simulink library "Garbage"  (R2023a)
settings: Solver=ode45  StartTime=0.0  StopTime=10.0  RelTol=1e-3  EnableMultiTasking=on
undefined symbols (not defined in the file or in its folder): c, ending_column_index, ending_row_index, framebuffer_16x1, framebuffer_2x2, framebuffer_4x4, framebuffer_8x8, HEIGHT, hot, POS_X, POS_Y, starting_column_index, starting_row_index, WIDTH
libraries: Memory_Blocks_Lib x459, simulink x235, simulink_extras x132, dspmtrx3 x116, Bit_Multiplexers_Lib x67, Bit_Demultiplexers_Lib x49, Byte_Multiplexers_Lib x40, Miscellaneous x38, Computer_Library x17, Decoders_Lib x14, commutil2 x12, Arithmetic_Operations_Lib x8
(part 24 of 26)

== system Garbage/Text_Display_1x16/Character_Table/Characters(ARROW_DOWN..RECTANGLE) ==
  Address_3_In(1b) : Inport
  Address_2_In(1b) : Inport  Port=2
  Address_1_In(1b) : Inport  Port=3
  Address_0_In(1b) : Inport  Port=4
  Byte_Multiplexer_16_to_1(Byte_0) : Byte_Multiplexers_Lib/Byte_Multiplexers_Lib/Byte_Multiplexer_16_to_1
  Byte_Multiplexer_16_to_1(Byte_1) : Byte_Multiplexers_Lib/Byte_Multiplexers_Lib/Byte_Multiplexer_16_to_1
  Byte_Multiplexer_16_to_1(Byte_2) : Byte_Multiplexers_Lib/Byte_Multiplexers_Lib/Byte_Multiplexer_16_to_1
  Byte_Multiplexer_16_to_1(Byte_3) : Byte_Multiplexers_Lib/Byte_Multiplexers_Lib/Byte_Multiplexer_16_to_1
  Byte_Multiplexer_16_to_1(Byte_4) : Byte_Multiplexers_Lib/Byte_Multiplexers_Lib/Byte_Multiplexer_16_to_1
  Byte_Multiplexer_16_to_1(Byte_5) : Byte_Multiplexers_Lib/Byte_Multiplexers_Lib/Byte_Multiplexer_16_to_1
  Byte_Multiplexer_16_to_1(Byte_6) : Byte_Multiplexers_Lib/Byte_Multiplexers_Lib/Byte_Multiplexer_16_to_1
  Byte_Multiplexer_16_to_1(Byte_7) : Byte_Multiplexers_Lib/Byte_Multiplexers_Lib/Byte_Multiplexer_16_to_1
  Char_ARROW_DOWN : Subsystem
  Char_ARROW_LEFT : Subsystem
  Char_ARROW_RIGHT : Subsystem
  Char_COMMA : Subsystem
  Char_CURSOR : Subsystem
  Char_DOT : Subsystem
  Char_EXCLAMATION_MARK : Subsystem
  Char_LEFT_PARENTHESES : Subsystem
  Char_PERCENT : Subsystem
  Char_QUESTION_MARK : Subsystem
  Char_QUOTE : Subsystem
  Char_RECTANGLE : Subsystem
  Char_RIGHT_PARENTHESES : Subsystem
  Char_SHARP : Subsystem
  Char_SPACE : Subsystem
  Char_UNDERSCORE : Subsystem
  Row_7(1B) : Outport
  Row_6(1B) : Outport  Port=2
  Row_5(1B) : Outport  Port=3
  Row_4(1B) : Outport  Port=4
  Row_3(1B) : Outport  Port=5
  Row_2(1B) : Outport  Port=6
  Row_1(1B) : Outport  Port=7
  Row_0(1B) : Outport  Port=8
  wires:
  Address_3_In(1b)/1 -> Byte_Multiplexer_16_to_1(Byte_0)/1, Byte_Multiplexer_16_to_1(Byte_1)/1, Byte_Multiplexer_16_to_1(Byte_2)/1, Byte_Multiplexer_16_to_1(Byte_3)/1, Byte_Multiplexer_16_to_1(Byte_4)/1, Byte_Multiplexer_16_to_1(Byte_5)/1, Byte_Multiplexer_16_to_1(Byte_6)/1, Byte_Multiplexer_16_to_1(Byte_7)/1
  Address_2_In(1b)/1 -> Byte_Multiplexer_16_to_1(Byte_0)/2, Byte_Multiplexer_16_to_1(Byte_1)/2, Byte_Multiplexer_16_to_1(Byte_2)/2, Byte_Multiplexer_16_to_1(Byte_3)/2, Byte_Multiplexer_16_to_1(Byte_4)/2, Byte_Multiplexer_16_to_1(Byte_5)/2, Byte_Multiplexer_16_to_1(Byte_6)/2, Byte_Multiplexer_16_to_1(Byte_7)/2
  Address_1_In(1b)/1 -> Byte_Multiplexer_16_to_1(Byte_0)/3, Byte_Multiplexer_16_to_1(Byte_1)/3, Byte_Multiplexer_16_to_1(Byte_2)/3, Byte_Multiplexer_16_to_1(Byte_3)/3, Byte_Multiplexer_16_to_1(Byte_4)/3, Byte_Multiplexer_16_to_1(Byte_5)/3, Byte_Multiplexer_16_to_1(Byte_6)/3, Byte_Multiplexer_16_to_1(Byte_7)/3
  Address_0_In(1b)/1 -> Byte_Multiplexer_16_to_1(Byte_0)/4, Byte_Multiplexer_16_to_1(Byte_1)/4, Byte_Multiplexer_16_to_1(Byte_2)/4, Byte_Multiplexer_16_to_1(Byte_3)/4, Byte_Multiplexer_16_to_1(Byte_4)/4, Byte_Multiplexer_16_to_1(Byte_5)/4, Byte_Multiplexer_16_to_1(Byte_6)/4, Byte_Multiplexer_16_to_1(Byte_7)/4
  Byte_Multiplexer_16_to_1(Byte_0)/1 -> Row_0(1B)/1
  Byte_Multiplexer_16_to_1(Byte_1)/1 -> Row_1(1B)/1
  Byte_Multiplexer_16_to_1(Byte_2)/1 -> Row_2(1B)/1
  Byte_Multiplexer_16_to_1(Byte_3)/1 -> Row_3(1B)/1
  Byte_Multiplexer_16_to_1(Byte_4)/1 -> Row_4(1B)/1
  Byte_Multiplexer_16_to_1(Byte_5)/1 -> Row_5(1B)/1
  Byte_Multiplexer_16_to_1(Byte_6)/1 -> Row_6(1B)/1
  Byte_Multiplexer_16_to_1(Byte_7)/1 -> Row_7(1B)/1
  Char_RECTANGLE/8(Row_0(1B)) -> Byte_Multiplexer_16_to_1(Byte_0)/5
  Char_CURSOR/8(Row_0(1B)) -> Byte_Multiplexer_16_to_1(Byte_0)/6
  Char_SPACE/8(Row_0(1B)) -> Byte_Multiplexer_16_to_1(Byte_0)/7
  Char_RIGHT_PARENTHESES/8(Row_0(1B)) -> Byte_Multiplexer_16_to_1(Byte_0)/8
  Char_LEFT_PARENTHESES/8(Row_0(1B)) -> Byte_Multiplexer_16_to_1(Byte_0)/9
  Char_PERCENT/8(Row_0(1B)) -> Byte_Multiplexer_16_to_1(Byte_0)/10
  Char_SHARP/8(Row_0(1B)) -> Byte_Multiplexer_16_to_1(Byte_0)/11
  Char_QUOTE/8(Row_0(1B)) -> Byte_Multiplexer_16_to_1(Byte_0)/12
  Char_EXCLAMATION_MARK/8(Row_0(1B)) -> Byte_Multiplexer_16_to_1(Byte_0)/13
  Char_QUESTION_MARK/8(Row_0(1B)) -> Byte_Multiplexer_16_to_1(Byte_0)/14
  Char_COMMA/8(Row_0(1B)) -> Byte_Multiplexer_16_to_1(Byte_0)/15
  Char_DOT/8(Row_0(1B)) -> Byte_Multiplexer_16_to_1(Byte_0)/16
  Char_UNDERSCORE/8(Row_0(1B)) -> Byte_Multiplexer_16_to_1(Byte_0)/17
  Char_ARROW_RIGHT/8(Row_0(1B)) -> Byte_Multiplexer_16_to_1(Byte_0)/18
  Char_ARROW_LEFT/8(Row_0(1B)) -> Byte_Multiplexer_16_to_1(Byte_0)/19
  Char_ARROW_DOWN/8(Row_0(1B)) -> Byte_Multiplexer_16_to_1(Byte_0)/20
  Char_RECTANGLE/7(Row_1(1B)) -> Byte_Multiplexer_16_to_1(Byte_1)/5
  Char_CURSOR/7(Row_1(1B)) -> Byte_Multiplexer_16_to_1(Byte_1)/6
  Char_SPACE/7(Row_1(1B)) -> Byte_Multiplexer_16_to_1(Byte_1)/7
  Char_LEFT_PARENTHESES/7(Row_1(1B)) -> Byte_Multiplexer_16_to_1(Byte_1)/9
  Char_PERCENT/7(Row_1(1B)) -> Byte_Multiplexer_16_to_1(Byte_1)/10
  Char_SHARP/7(Row_1(1B)) -> Byte_Multiplexer_16_to_1(Byte_1)/11
  Char_QUOTE/7(Row_1(1B)) -> Byte_Multiplexer_16_to_1(Byte_1)/12
  Char_EXCLAMATION_MARK/7(Row_1(1B)) -> Byte_Multiplexer_16_to_1(Byte_1)/13
  Char_QUESTION_MARK/7(Row_1(1B)) -> Byte_Multiplexer_16_to_1(Byte_1)/14
  Char_COMMA/7(Row_1(1B)) -> Byte_Multiplexer_16_to_1(Byte_1)/15
  Char_DOT/7(Row_1(1B)) -> Byte_Multiplexer_16_to_1(Byte_1)/16
  Char_UNDERSCORE/7(Row_1(1B)) -> Byte_Multiplexer_16_to_1(Byte_1)/17
  Char_ARROW_RIGHT/7(Row_1(1B)) -> Byte_Multiplexer_16_to_1(Byte_1)/18
  Char_ARROW_LEFT/7(Row_1(1B)) -> Byte_Multiplexer_16_to_1(Byte_1)/19
  Char_ARROW_DOWN/7(Row_1(1B)) -> Byte_Multiplexer_16_to_1(Byte_1)/20
  Char_RECTANGLE/6(Row_2(1B)) -> Byte_Multiplexer_16_to_1(Byte_2)/5
  Char_CURSOR/6(Row_2(1B)) -> Byte_Multiplexer_16_to_1(Byte_2)/6
  Char_SPACE/6(Row_2(1B)) -> Byte_Multiplexer_16_to_1(Byte_2)/7
  Char_RIGHT_PARENTHESES/6(Row_2(1B)) -> Byte_Multiplexer_16_to_1(Byte_2)/8
  Char_RIGHT_PARENTHESES/7(Row_1(1B)) -> Byte_Multiplexer_16_to_1(Byte_1)/8
  Char_LEFT_PARENTHESES/6(Row_2(1B)) -> Byte_Multiplexer_16_to_1(Byte_2)/9
  Char_PERCENT/6(Row_2(1B)) -> Byte_Multiplexer_16_to_1(Byte_2)/10
  Char_SHARP/6(Row_2(1B)) -> Byte_Multiplexer_16_to_1(Byte_2)/11
  Char_QUOTE/6(Row_2(1B)) -> Byte_Multiplexer_16_to_1(Byte_2)/12
  Char_EXCLAMATION_MARK/6(Row_2(1B)) -> Byte_Multiplexer_16_to_1(Byte_2)/13
  Char_QUESTION_MARK/6(Row_2(1B)) -> Byte_Multiplexer_16_to_1(Byte_2)/14
  Char_COMMA/6(Row_2(1B)) -> Byte_Multiplexer_16_to_1(Byte_2)/15
  Char_DOT/6(Row_2(1B)) -> Byte_Multiplexer_16_to_1(Byte_2)/16
  Char_UNDERSCORE/6(Row_2(1B)) -> Byte_Multiplexer_16_to_1(Byte_2)/17
  Char_ARROW_RIGHT/6(Row_2(1B)) -> Byte_Multiplexer_16_to_1(Byte_2)/18
  Char_ARROW_LEFT/6(Row_2(1B)) -> Byte_Multiplexer_16_to_1(Byte_2)/19
  Char_ARROW_DOWN/6(Row_2(1B)) -> Byte_Multiplexer_16_to_1(Byte_2)/20
  Char_RECTANGLE/5(Row_3(1B)) -> Byte_Multiplexer_16_to_1(Byte_3)/5
  Char_CURSOR/5(Row_3(1B)) -> Byte_Multiplexer_16_to_1(Byte_3)/6
  Char_SPACE/5(Row_3(1B)) -> Byte_Multiplexer_16_to_1(Byte_3)/7
  Char_RIGHT_PARENTHESES/5(Row_3(1B)) -> Byte_Multiplexer_16_to_1(Byte_3)/8
  Char_LEFT_PARENTHESES/5(Row_3(1B)) -> Byte_Multiplexer_16_to_1(Byte_3)/9
  Char_PERCENT/5(Row_3(1B)) -> Byte_Multiplexer_16_to_1(Byte_3)/10
  Char_SHARP/5(Row_3(1B)) -> Byte_Multiplexer_16_to_1(Byte_3)/11
  Char_QUOTE/5(Row_3(1B)) -> Byte_Multiplexer_16_to_1(Byte_3)/12
  Char_QUESTION_MARK/5(Row_3(1B)) -> Byte_Multiplexer_16_to_1(Byte_3)/14
  Char_EXCLAMATION_MARK/5(Row_3(1B)) -> Byte_Multiplexer_16_to_1(Byte_3)/13
  Char_COMMA/5(Row_3(1B)) -> Byte_Multiplexer_16_to_1(Byte_3)/15
  Char_DOT/5(Row_3(1B)) -> Byte_Multiplexer_16_to_1(Byte_3)/16
  Char_UNDERSCORE/5(Row_3(1B)) -> Byte_Multiplexer_16_to_1(Byte_3)/17
  Char_ARROW_RIGHT/5(Row_3(1B)) -> Byte_Multiplexer_16_to_1(Byte_3)/18
  Char_ARROW_LEFT/5(Row_3(1B)) -> Byte_Multiplexer_16_to_1(Byte_3)/19
  Char_ARROW_DOWN/5(Row_3(1B)) -> Byte_Multiplexer_16_to_1(Byte_3)/20
  Char_RECTANGLE/4(Row_4(1B)) -> Byte_Multiplexer_16_to_1(Byte_4)/5
  Char_CURSOR/4(Row_4(1B)) -> Byte_Multiplexer_16_to_1(Byte_4)/6
  Char_SPACE/4(Row_4(1B)) -> Byte_Multiplexer_16_to_1(Byte_4)/7
  Char_RIGHT_PARENTHESES/4(Row_4(1B)) -> Byte_Multiplexer_16_to_1(Byte_4)/8
  Char_LEFT_PARENTHESES/4(Row_4(1B)) -> Byte_Multiplexer_16_to_1(Byte_4)/9
  Char_PERCENT/4(Row_4(1B)) -> Byte_Multiplexer_16_to_1(Byte_4)/10
  Char_SHARP/4(Row_4(1B)) -> Byte_Multiplexer_16_to_1(Byte_4)/11
  Char_QUOTE/4(Row_4(1B)) -> Byte_Multiplexer_16_to_1(Byte_4)/12
  Char_EXCLAMATION_MARK/4(Row_4(1B)) -> Byte_Multiplexer_16_to_1(Byte_4)/13
  Char_QUESTION_MARK/4(Row_4(1B)) -> Byte_Multiplexer_16_to_1(Byte_4)/14
  Char_COMMA/4(Row_4(1B)) -> Byte_Multiplexer_16_to_1(Byte_4)/15
  Char_DOT/4(Row_4(1B)) -> Byte_Multiplexer_16_to_1(Byte_4)/16
  Char_UNDERSCORE/4(Row_4(1B)) -> Byte_Multiplexer_16_to_1(Byte_4)/17
  Char_ARROW_RIGHT/4(Row_4(1B)) -> Byte_Multiplexer_16_to_1(Byte_4)/18
  Char_ARROW_LEFT/4(Row_4(1B)) -> Byte_Multiplexer_16_to_1(Byte_4)/19
  Char_ARROW_DOWN/4(Row_4(1B)) -> Byte_Multiplexer_16_to_1(Byte_4)/20
  Char_RECTANGLE/3(Row_5(1B)) -> Byte_Multiplexer_16_to_1(Byte_5)/5
  Char_CURSOR/3(Row_5(1B)) -> Byte_Multiplexer_16_to_1(Byte_5)/6
  Char_SPACE/3(Row_5(1B)) -> Byte_Multiplexer_16_to_1(Byte_5)/7
  Char_RIGHT_PARENTHESES/3(Row_5(1B)) -> Byte_Multiplexer_16_to_1(Byte_5)/8
  Char_LEFT_PARENTHESES/3(Row_5(1B)) -> Byte_Multiplexer_16_to_1(Byte_5)/9
  Char_PERCENT/3(Row_5(1B)) -> Byte_Multiplexer_16_to_1(Byte_5)/10
  Char_SHARP/3(Row_5(1B)) -> Byte_Multiplexer_16_to_1(Byte_5)/11
  Char_QUOTE/3(Row_5(1B)) -> Byte_Multiplexer_16_to_1(Byte_5)/12
  Char_EXCLAMATION_MARK/3(Row_5(1B)) -> Byte_Multiplexer_16_to_1(Byte_5)/13
  Char_QUESTION_MARK/3(Row_5(1B)) -> Byte_Multiplexer_16_to_1(Byte_5)/14
  Char_COMMA/3(Row_5(1B)) -> Byte_Multiplexer_16_to_1(Byte_5)/15
  Char_DOT/3(Row_5(1B)) -> Byte_Multiplexer_16_to_1(Byte_5)/16
  Char_UNDERSCORE/3(Row_5(1B)) -> Byte_Multiplexer_16_to_1(Byte_5)/17
  Char_ARROW_RIGHT/3(Row_5(1B)) -> Byte_Multiplexer_16_to_1(Byte_5)/18
  Char_ARROW_LEFT/3(Row_5(1B)) -> Byte_Multiplexer_16_to_1(Byte_5)/19
  Char_ARROW_DOWN/3(Row_5(1B)) -> Byte_Multiplexer_16_to_1(Byte_5)/20
  Char_CURSOR/2(Row_6(1B)) -> Byte_Multiplexer_16_to_1(Byte_6)/6
  Char_SPACE/2(Row_6(1B)) -> Byte_Multiplexer_16_to_1(Byte_6)/7
  Char_RIGHT_PARENTHESES/2(Row_6(1B)) -> Byte_Multiplexer_16_to_1(Byte_6)/8
  Char_LEFT_PARENTHESES/2(Row_6(1B)) -> Byte_Multiplexer_16_to_1(Byte_6)/9
  Char_PERCENT/2(Row_6(1B)) -> Byte_Multiplexer_16_to_1(Byte_6)/10
  Char_SHARP/2(Row_6(1B)) -> Byte_Multiplexer_16_to_1(Byte_6)/11
  Char_QUOTE/2(Row_6(1B)) -> Byte_Multiplexer_16_to_1(Byte_6)/12
  Char_EXCLAMATION_MARK/2(Row_6(1B)) -> Byte_Multiplexer_16_to_1(Byte_6)/13
  Char_QUESTION_MARK/2(Row_6(1B)) -> Byte_Multiplexer_16_to_1(Byte_6)/14
  Char_COMMA/2(Row_6(1B)) -> Byte_Multiplexer_16_to_1(Byte_6)/15
  Char_DOT/2(Row_6(1B)) -> Byte_Multiplexer_16_to_1(Byte_6)/16
  Char_UNDERSCORE/2(Row_6(1B)) -> Byte_Multiplexer_16_to_1(Byte_6)/17
  Char_ARROW_RIGHT/2(Row_6(1B)) -> Byte_Multiplexer_16_to_1(Byte_6)/18
  Char_ARROW_LEFT/2(Row_6(1B)) -> Byte_Multiplexer_16_to_1(Byte_6)/19
  Char_ARROW_DOWN/2(Row_6(1B)) -> Byte_Multiplexer_16_to_1(Byte_6)/20
  Char_RECTANGLE/2(Row_6(1B)) -> Byte_Multiplexer_16_to_1(Byte_6)/5
  Char_ARROW_DOWN/1(Row_7(1B)) -> Byte_Multiplexer_16_to_1(Byte_7)/20
  Char_ARROW_LEFT/1(Row_7(1B)) -> Byte_Multiplexer_16_to_1(Byte_7)/19
  Char_ARROW_RIGHT/1(Row_7(1B)) -> Byte_Multiplexer_16_to_1(Byte_7)/18
  Char_UNDERSCORE/1(Row_7(1B)) -> Byte_Multiplexer_16_to_1(Byte_7)/17
  Char_DOT/1(Row_7(1B)) -> Byte_Multiplexer_16_to_1(Byte_7)/16
  Char_COMMA/1(Row_7(1B)) -> Byte_Multiplexer_16_to_1(Byte_7)/15
  Char_QUESTION_MARK/1(Row_7(1B)) -> Byte_Multiplexer_16_to_1(Byte_7)/14
  Char_EXCLAMATION_MARK/1(Row_7(1B)) -> Byte_Multiplexer_16_to_1(Byte_7)/13
  Char_QUOTE/1(Row_7(1B)) -> Byte_Multiplexer_16_to_1(Byte_7)/12
  Char_SHARP/1(Row_7(1B)) -> Byte_Multiplexer_16_to_1(Byte_7)/11
  Char_PERCENT/1(Row_7(1B)) -> Byte_Multiplexer_16_to_1(Byte_7)/10
  Char_LEFT_PARENTHESES/1(Row_7(1B)) -> Byte_Multiplexer_16_to_1(Byte_7)/9
  Char_RIGHT_PARENTHESES/1(Row_7(1B)) -> Byte_Multiplexer_16_to_1(Byte_7)/8
  Char_SPACE/1(Row_7(1B)) -> Byte_Multiplexer_16_to_1(Byte_7)/7
  Char_CURSOR/1(Row_7(1B)) -> Byte_Multiplexer_16_to_1(Byte_7)/6
  Char_RECTANGLE/1(Row_7(1B)) -> Byte_Multiplexer_16_to_1(Byte_7)/5

== system Garbage/Text_Display_1x16/Character_Table/Characters(G..W) ==
  Address_3_In(1b) : Inport
  Address_2_In(1b) : Inport  Port=2
  Address_1_In(1b) : Inport  Port=3
  Address_0_In(1b) : Inport  Port=4
  Byte_Multiplexer_16_to_1(Byte_0) : Byte_Multiplexers_Lib/Byte_Multiplexers_Lib/Byte_Multiplexer_16_to_1
  Byte_Multiplexer_16_to_1(Byte_1) : Byte_Multiplexers_Lib/Byte_Multiplexers_Lib/Byte_Multiplexer_16_to_1
  Byte_Multiplexer_16_to_1(Byte_2) : Byte_Multiplexers_Lib/Byte_Multiplexers_Lib/Byte_Multiplexer_16_to_1
  Byte_Multiplexer_16_to_1(Byte_3) : Byte_Multiplexers_Lib/Byte_Multiplexers_Lib/Byte_Multiplexer_16_to_1
  Byte_Multiplexer_16_to_1(Byte_4) : Byte_Multiplexers_Lib/Byte_Multiplexers_Lib/Byte_Multiplexer_16_to_1
  Byte_Multiplexer_16_to_1(Byte_5) : Byte_Multiplexers_Lib/Byte_Multiplexers_Lib/Byte_Multiplexer_16_to_1
  Byte_Multiplexer_16_to_1(Byte_6) : Byte_Multiplexers_Lib/Byte_Multiplexers_Lib/Byte_Multiplexer_16_to_1
  Byte_Multiplexer_16_to_1(Byte_7) : Byte_Multiplexers_Lib/Byte_Multiplexers_Lib/Byte_Multiplexer_16_to_1
  Char_G : Subsystem
  Char_H : Subsystem
  Char_I : Subsystem
  Char_J : Subsystem
  Char_K : Subsystem
  Char_L : Subsystem
  Char_M : Subsystem
  Char_N : Subsystem
  Char_O : Subsystem
  Char_P : Subsystem
  Char_R : Subsystem
  Char_S : Subsystem
  Char_T : Subsystem
  Char_U : Subsystem
  Char_V : Subsystem
  Char_W : Subsystem
  Row_7(1B) : Outport
  Row_6(1B) : Outport  Port=2
  Row_5(1B) : Outport  Port=3
  Row_4(1B) : Outport  Port=4
  Row_3(1B) : Outport  Port=5
  Row_2(1B) : Outport  Port=6
  Row_1(1B) : Outport  Port=7
  Row_0(1B) : Outport  Port=8
  wires:
  Address_3_In(1b)/1 -> Byte_Multiplexer_16_to_1(Byte_0)/1, Byte_Multiplexer_16_to_1(Byte_1)/1, Byte_Multiplexer_16_to_1(Byte_2)/1, Byte_Multiplexer_16_to_1(Byte_3)/1, Byte_Multiplexer_16_to_1(Byte_4)/1, Byte_Multiplexer_16_to_1(Byte_5)/1, Byte_Multiplexer_16_to_1(Byte_6)/1, Byte_Multiplexer_16_to_1(Byte_7)/1
  Address_2_In(1b)/1 -> Byte_Multiplexer_16_to_1(Byte_0)/2, Byte_Multiplexer_16_to_1(Byte_1)/2, Byte_Multiplexer_16_to_1(Byte_2)/2, Byte_Multiplexer_16_to_1(Byte_3)/2, Byte_Multiplexer_16_to_1(Byte_4)/2, Byte_Multiplexer_16_to_1(Byte_5)/2, Byte_Multiplexer_16_to_1(Byte_6)/2, Byte_Multiplexer_16_to_1(Byte_7)/2
  Address_1_In(1b)/1 -> Byte_Multiplexer_16_to_1(Byte_0)/3, Byte_Multiplexer_16_to_1(Byte_1)/3, Byte_Multiplexer_16_to_1(Byte_2)/3, Byte_Multiplexer_16_to_1(Byte_3)/3, Byte_Multiplexer_16_to_1(Byte_4)/3, Byte_Multiplexer_16_to_1(Byte_5)/3, Byte_Multiplexer_16_to_1(Byte_6)/3, Byte_Multiplexer_16_to_1(Byte_7)/3
  Address_0_In(1b)/1 -> Byte_Multiplexer_16_to_1(Byte_0)/4, Byte_Multiplexer_16_to_1(Byte_1)/4, Byte_Multiplexer_16_to_1(Byte_2)/4, Byte_Multiplexer_16_to_1(Byte_3)/4, Byte_Multiplexer_16_to_1(Byte_4)/4, Byte_Multiplexer_16_to_1(Byte_5)/4, Byte_Multiplexer_16_to_1(Byte_6)/4, Byte_Multiplexer_16_to_1(Byte_7)/4
  Byte_Multiplexer_16_to_1(Byte_0)/1 -> Row_0(1B)/1
  Byte_Multiplexer_16_to_1(Byte_1)/1 -> Row_1(1B)/1
  Byte_Multiplexer_16_to_1(Byte_2)/1 -> Row_2(1B)/1
  Byte_Multiplexer_16_to_1(Byte_3)/1 -> Row_3(1B)/1
  Byte_Multiplexer_16_to_1(Byte_4)/1 -> Row_4(1B)/1
  Byte_Multiplexer_16_to_1(Byte_5)/1 -> Row_5(1B)/1
  Byte_Multiplexer_16_to_1(Byte_6)/1 -> Row_6(1B)/1
  Byte_Multiplexer_16_to_1(Byte_7)/1 -> Row_7(1B)/1
  Char_W/8(Row_0(1B)) -> Byte_Multiplexer_16_to_1(Byte_0)/5
  Char_V/8(Row_0(1B)) -> Byte_Multiplexer_16_to_1(Byte_0)/6
  Char_U/8(Row_0(1B)) -> Byte_Multiplexer_16_to_1(Byte_0)/7
  Char_T/8(Row_0(1B)) -> Byte_Multiplexer_16_to_1(Byte_0)/8
  Char_S/8(Row_0(1B)) -> Byte_Multiplexer_16_to_1(Byte_0)/9
  Char_R/8(Row_0(1B)) -> Byte_Multiplexer_16_to_1(Byte_0)/10
  Char_P/8(Row_0(1B)) -> Byte_Multiplexer_16_to_1(Byte_0)/11
  Char_O/8(Row_0(1B)) -> Byte_Multiplexer_16_to_1(Byte_0)/12
  Char_N/8(Row_0(1B)) -> Byte_Multiplexer_16_to_1(Byte_0)/13
  Char_M/8(Row_0(1B)) -> Byte_Multiplexer_16_to_1(Byte_0)/14
  Char_L/8(Row_0(1B)) -> Byte_Multiplexer_16_to_1(Byte_0)/15
  Char_K/8(Row_0(1B)) -> Byte_Multiplexer_16_to_1(Byte_0)/16
  Char_J/8(Row_0(1B)) -> Byte_Multiplexer_16_to_1(Byte_0)/17
  Char_I/8(Row_0(1B)) -> Byte_Multiplexer_16_to_1(Byte_0)/18
  Char_H/8(Row_0(1B)) -> Byte_Multiplexer_16_to_1(Byte_0)/19
  Char_G/8(Row_0(1B)) -> Byte_Multiplexer_16_to_1(Byte_0)/20
  Char_W/7(Row_1(1B)) -> Byte_Multiplexer_16_to_1(Byte_1)/5
  Char_V/7(Row_1(1B)) -> Byte_Multiplexer_16_to_1(Byte_1)/6
  Char_U/7(Row_1(1B)) -> Byte_Multiplexer_16_to_1(Byte_1)/7
  Char_S/7(Row_1(1B)) -> Byte_Multiplexer_16_to_1(Byte_1)/9
  Char_R/7(Row_1(1B)) -> Byte_Multiplexer_16_to_1(Byte_1)/10
  Char_P/7(Row_1(1B)) -> Byte_Multiplexer_16_to_1(Byte_1)/11
  Char_O/7(Row_1(1B)) -> Byte_Multiplexer_16_to_1(Byte_1)/12
  Char_N/7(Row_1(1B)) -> Byte_Multiplexer_16_to_1(Byte_1)/13
  Char_M/7(Row_1(1B)) -> Byte_Multiplexer_16_to_1(Byte_1)/14
  Char_L/7(Row_1(1B)) -> Byte_Multiplexer_16_to_1(Byte_1)/15
  Char_K/7(Row_1(1B)) -> Byte_Multiplexer_16_to_1(Byte_1)/16
  Char_J/7(Row_1(1B)) -> Byte_Multiplexer_16_to_1(Byte_1)/17
  Char_I/7(Row_1(1B)) -> Byte_Multiplexer_16_to_1(Byte_1)/18
  Char_H/7(Row_1(1B)) -> Byte_Multiplexer_16_to_1(Byte_1)/19
  Char_G/7(Row_1(1B)) -> Byte_Multiplexer_16_to_1(Byte_1)/20
  Char_W/6(Row_2(1B)) -> Byte_Multiplexer_16_to_1(Byte_2)/5
  Char_V/6(Row_2(1B)) -> Byte_Multiplexer_16_to_1(Byte_2)/6
  Char_U/6(Row_2(1B)) -> Byte_Multiplexer_16_to_1(Byte_2)/7
  Char_T/6(Row_2(1B)) -> Byte_Multiplexer_16_to_1(Byte_2)/8
  Char_T/7(Row_1(1B)) -> Byte_Multiplexer_16_to_1(Byte_1)/8
  Char_S/6(Row_2(1B)) -> Byte_Multiplexer_16_to_1(Byte_2)/9
  Char_R/6(Row_2(1B)) -> Byte_Multiplexer_16_to_1(Byte_2)/10
  Char_P/6(Row_2(1B)) -> Byte_Multiplexer_16_to_1(Byte_2)/11
  Char_O/6(Row_2(1B)) -> Byte_Multiplexer_16_to_1(Byte_2)/12
  Char_N/6(Row_2(1B)) -> Byte_Multiplexer_16_to_1(Byte_2)/13
  Char_M/6(Row_2(1B)) -> Byte_Multiplexer_16_to_1(Byte_2)/14
  Char_L/6(Row_2(1B)) -> Byte_Multiplexer_16_to_1(Byte_2)/15
  Char_K/6(Row_2(1B)) -> Byte_Multiplexer_16_to_1(Byte_2)/16
  Char_J/6(Row_2(1B)) -> Byte_Multiplexer_16_to_1(Byte_2)/17
  Char_I/6(Row_2(1B)) -> Byte_Multiplexer_16_to_1(Byte_2)/18
  Char_H/6(Row_2(1B)) -> Byte_Multiplexer_16_to_1(Byte_2)/19
  Char_G/6(Row_2(1B)) -> Byte_Multiplexer_16_to_1(Byte_2)/20
  Char_W/5(Row_3(1B)) -> Byte_Multiplexer_16_to_1(Byte_3)/5
  Char_V/5(Row_3(1B)) -> Byte_Multiplexer_16_to_1(Byte_3)/6
  Char_U/5(Row_3(1B)) -> Byte_Multiplexer_16_to_1(Byte_3)/7
  Char_T/5(Row_3(1B)) -> Byte_Multiplexer_16_to_1(Byte_3)/8
  Char_S/5(Row_3(1B)) -> Byte_Multiplexer_16_to_1(Byte_3)/9
  Char_R/5(Row_3(1B)) -> Byte_Multiplexer_16_to_1(Byte_3)/10
  Char_P/5(Row_3(1B)) -> Byte_Multiplexer_16_to_1(Byte_3)/11
  Char_O/5(Row_3(1B)) -> Byte_Multiplexer_16_to_1(Byte_3)/12
  Char_M/5(Row_3(1B)) -> Byte_Multiplexer_16_to_1(Byte_3)/14
  Char_N/5(Row_3(1B)) -> Byte_Multiplexer_16_to_1(Byte_3)/13
  Char_L/5(Row_3(1B)) -> Byte_Multiplexer_16_to_1(Byte_3)/15
  Char_K/5(Row_3(1B)) -> Byte_Multiplexer_16_to_1(Byte_3)/16
  Char_J/5(Row_3(1B)) -> Byte_Multiplexer_16_to_1(Byte_3)/17
  Char_I/5(Row_3(1B)) -> Byte_Multiplexer_16_to_1(Byte_3)/18
  Char_H/5(Row_3(1B)) -> Byte_Multiplexer_16_to_1(Byte_3)/19
  Char_G/5(Row_3(1B)) -> Byte_Multiplexer_16_to_1(Byte_3)/20
  Char_W/4(Row_4(1B)) -> Byte_Multiplexer_16_to_1(Byte_4)/5
  Char_V/4(Row_4(1B)) -> Byte_Multiplexer_16_to_1(Byte_4)/6
  Char_U/4(Row_4(1B)) -> Byte_Multiplexer_16_to_1(Byte_4)/7
  Char_T/4(Row_4(1B)) -> Byte_Multiplexer_16_to_1(Byte_4)/8
  Char_S/4(Row_4(1B)) -> Byte_Multiplexer_16_to_1(Byte_4)/9
  Char_R/4(Row_4(1B)) -> Byte_Multiplexer_16_to_1(Byte_4)/10
  Char_P/4(Row_4(1B)) -> Byte_Multiplexer_16_to_1(Byte_4)/11
  Char_O/4(Row_4(1B)) -> Byte_Multiplexer_16_to_1(Byte_4)/12
  Char_N/4(Row_4(1B)) -> Byte_Multiplexer_16_to_1(Byte_4)/13
  Char_M/4(Row_4(1B)) -> Byte_Multiplexer_16_to_1(Byte_4)/14
  Char_L/4(Row_4(1B)) -> Byte_Multiplexer_16_to_1(Byte_4)/15
  Char_K/4(Row_4(1B)) -> Byte_Multiplexer_16_to_1(Byte_4)/16
  Char_J/4(Row_4(1B)) -> Byte_Multiplexer_16_to_1(Byte_4)/17
  Char_I/4(Row_4(1B)) -> Byte_Multiplexer_16_to_1(Byte_4)/18
  Char_H/4(Row_4(1B)) -> Byte_Multiplexer_16_to_1(Byte_4)/19
  Char_G/4(Row_4(1B)) -> Byte_Multiplexer_16_to_1(Byte_4)/20
  Char_W/3(Row_5(1B)) -> Byte_Multiplexer_16_to_1(Byte_5)/5
  Char_V/3(Row_5(1B)) -> Byte_Multiplexer_16_to_1(Byte_5)/6
  Char_U/3(Row_5(1B)) -> Byte_Multiplexer_16_to_1(Byte_5)/7
  Char_T/3(Row_5(1B)) -> Byte_Multiplexer_16_to_1(Byte_5)/8
  Char_S/3(Row_5(1B)) -> Byte_Multiplexer_16_to_1(Byte_5)/9
  Char_R/3(Row_5(1B)) -> Byte_Multiplexer_16_to_1(Byte_5)/10
  Char_P/3(Row_5(1B)) -> Byte_Multiplexer_16_to_1(Byte_5)/11
  Char_O/3(Row_5(1B)) -> Byte_Multiplexer_16_to_1(Byte_5)/12
  Char_N/3(Row_5(1B)) -> Byte_Multiplexer_16_to_1(Byte_5)/13
  Char_M/3(Row_5(1B)) -> Byte_Multiplexer_16_to_1(Byte_5)/14
  Char_L/3(Row_5(1B)) -> Byte_Multiplexer_16_to_1(Byte_5)/15
  Char_K/3(Row_5(1B)) -> Byte_Multiplexer_16_to_1(Byte_5)/16
  Char_J/3(Row_5(1B)) -> Byte_Multiplexer_16_to_1(Byte_5)/17
  Char_I/3(Row_5(1B)) -> Byte_Multiplexer_16_to_1(Byte_5)/18
  Char_H/3(Row_5(1B)) -> Byte_Multiplexer_16_to_1(Byte_5)/19
  Char_G/3(Row_5(1B)) -> Byte_Multiplexer_16_to_1(Byte_5)/20
  Char_V/2(Row_6(1B)) -> Byte_Multiplexer_16_to_1(Byte_6)/6
  Char_U/2(Row_6(1B)) -> Byte_Multiplexer_16_to_1(Byte_6)/7
  Char_T/2(Row_6(1B)) -> Byte_Multiplexer_16_to_1(Byte_6)/8
  Char_S/2(Row_6(1B)) -> Byte_Multiplexer_16_to_1(Byte_6)/9
  Char_R/2(Row_6(1B)) -> Byte_Multiplexer_16_to_1(Byte_6)/10
  Char_P/2(Row_6(1B)) -> Byte_Multiplexer_16_to_1(Byte_6)/11
  Char_O/2(Row_6(1B)) -> Byte_Multiplexer_16_to_1(Byte_6)/12
  Char_N/2(Row_6(1B)) -> Byte_Multiplexer_16_to_1(Byte_6)/13
  Char_M/2(Row_6(1B)) -> Byte_Multiplexer_16_to_1(Byte_6)/14
  Char_L/2(Row_6(1B)) -> Byte_Multiplexer_16_to_1(Byte_6)/15
  Char_K/2(Row_6(1B)) -> Byte_Multiplexer_16_to_1(Byte_6)/16
  Char_J/2(Row_6(1B)) -> Byte_Multiplexer_16_to_1(Byte_6)/17
  Char_I/2(Row_6(1B)) -> Byte_Multiplexer_16_to_1(Byte_6)/18
  Char_H/2(Row_6(1B)) -> Byte_Multiplexer_16_to_1(Byte_6)/19
  Char_G/2(Row_6(1B)) -> Byte_Multiplexer_16_to_1(Byte_6)/20
  Char_W/2(Row_6(1B)) -> Byte_Multiplexer_16_to_1(Byte_6)/5
  Char_G/1(Row_7(1B)) -> Byte_Multiplexer_16_to_1(Byte_7)/20
  Char_H/1(Row_7(1B)) -> Byte_Multiplexer_16_to_1(Byte_7)/19
  Char_I/1(Row_7(1B)) -> Byte_Multiplexer_16_to_1(Byte_7)/18
  Char_J/1(Row_7(1B)) -> Byte_Multiplexer_16_to_1(Byte_7)/17
  Char_K/1(Row_7(1B)) -> Byte_Multiplexer_16_to_1(Byte_7)/16
  Char_L/1(Row_7(1B)) -> Byte_Multiplexer_16_to_1(Byte_7)/15
  Char_M/1(Row_7(1B)) -> Byte_Multiplexer_16_to_1(Byte_7)/14
  Char_N/1(Row_7(1B)) -> Byte_Multiplexer_16_to_1(Byte_7)/13
  Char_O/1(Row_7(1B)) -> Byte_Multiplexer_16_to_1(Byte_7)/12
  Char_P/1(Row_7(1B)) -> Byte_Multiplexer_16_to_1(Byte_7)/11
  Char_R/1(Row_7(1B)) -> Byte_Multiplexer_16_to_1(Byte_7)/10
  Char_S/1(Row_7(1B)) -> Byte_Multiplexer_16_to_1(Byte_7)/9
  Char_T/1(Row_7(1B)) -> Byte_Multiplexer_16_to_1(Byte_7)/8
  Char_U/1(Row_7(1B)) -> Byte_Multiplexer_16_to_1(Byte_7)/7
  Char_V/1(Row_7(1B)) -> Byte_Multiplexer_16_to_1(Byte_7)/6
  Char_W/1(Row_7(1B)) -> Byte_Multiplexer_16_to_1(Byte_7)/5
